simulink model "MX_Gateway"  (Simulink 9.0)
settings: Solver=FixedStepDiscrete  StartTime=0.0  StopTime=100  FixedStep=0.01  RelTol=1e-3
values from neighbouring files (symbols this model uses):
  ACTIVE = Bobcat.Parameter; CreateDefine( ACTIVE                         ,1                          ,'Define' ,'defines.h' )    [defines.m]
  ACTIVE_MODE_ONE = Bobcat.Parameter; CreateDefine( ACTIVE_MODE_ONE                ,19                         ,'Define' ,'defines.h' )    [defines.m]
  AUTHENTICATED = Bobcat.Parameter; CreateDefine( AUTHENTICATED                  ,1                          ,'Define' ,'defines.h' )    [defines.m]
  AUX_EMEA_CONTROLS = Bobcat.Parameter; CreateDefine( AUX_EMEA_CONTROLS  ,1   ,'Define' ,'defines.h' )    [enums.m]
  AUX_FLOW_HIGH = Bobcat.Parameter; CreateDefine( AUX_FLOW_HIGH           ,4  ,'Define' ,'defines.h' )    [enums.m]
  AUX_FLOW_INACTIVE = Bobcat.Parameter; CreateDefine( AUX_FLOW_INACTIVE       ,0  ,'Define' ,'defines.h' )    [enums.m]
  AUX_FLOW_LOW = Bobcat.Parameter; CreateDefine( AUX_FLOW_LOW            ,1  ,'Define' ,'defines.h' )    [enums.m]
  AUX_FLOW_MEDIUM = Bobcat.Parameter; CreateDefine( AUX_FLOW_MEDIUM         ,2  ,'Define' ,'defines.h' )    [enums.m]
  AUX_FLOW_STANDARD = Bobcat.Parameter; CreateDefine( AUX_FLOW_STANDARD       ,3  ,'Define' ,'defines.h' )    [enums.m]
  AUX_NON_FUNCTIONAL = Bobcat.Parameter; CreateDefine( AUX_NON_FUNCTIONAL ,255 ,'Define' ,'defines.h' )    [enums.m]
  BASE_ACTIVE = Bobcat.Parameter; CreateDefine( BASE_ACTIVE                    ,253                        ,'Define' ,'defines.h' )    [defines.m]
  BASE_DETENT = Bobcat.Parameter; CreateDefine( BASE_DETENT                    ,1                          ,'Define' ,'defines.h' )    [defines.m]
  BEEP_NONE = Bobcat.Parameter; CreateDefine( BEEP_NONE                      ,255                        ,'Define' ,'defines.h' )    [defines.m]
  CD_AIR_FILTER_DEBOUNCE_NORMAL = Bobcat.Parameter; CreateAliasCD( CD_AIR_FILTER_DEBOUNCE_NORMAL                        ,uint16(500)                ,'ConstantDataPointer' ,'CONSTANT_DATA' ,'AIR_FILTER_DEBOUNCE_NORMAL')    [cdata.m]    (also in hc12_cdata.m; m4_cdata.m)
  CD_AIR_FILTER_DEBOUNCE_NOT_CONNECTED = Bobcat.Parameter; CreateAliasCD( CD_AIR_FILTER_DEBOUNCE_NOT_CONNECTED                 ,uint16(3000)               ,'ConstantDataPointer' ,'CONSTANT_DATA' ,'AIR_FILTER_DEBOUNCE_NOT_CONNECTED')    [cdata.m]    (also in hc12_cdata.m; m4_cdata.m)
  CD_AIR_FILTER_DEBOUNCE_PLUGGED = Bobcat.Parameter; CreateAliasCD( CD_AIR_FILTER_DEBOUNCE_PLUGGED                       ,uint16(3000)               ,'ConstantDataPointer' ,'CONSTANT_DATA' ,'AIR_FILTER_DEBOUNCE_PLUGGED')    [cdata.m]    (also in hc12_cdata.m; m4_cdata.m)
  CD_AIR_FILTER_FUNCTION = Bobcat.Parameter; CreateAliasCD( CD_AIR_FILTER_FUNCTION                               ,uint8(255)                 ,'ConstantDataPointer' ,'CONSTANT_DATA' ,'AIR_FILTER_FUNCTION')    [cdata.m]    (also in hc12_cdata.m; m4_cdata.m)
  CD_AIR_FILTER_PLUGGED_WEIGHT = Bobcat.Parameter; CreateAliasCD( CD_AIR_FILTER_PLUGGED_WEIGHT                         ,uint8(2)                   ,'ConstantDataPointer' ,'CONSTANT_DATA' ,'AIR_FILTER_PLUGGED_WEIGHT')    [cdata.m]    (also in hc12_cdata.m; m4_cdata.m)
  CD_AIR_FILTER_UNPLUGGED_WEIGHT = Bobcat.Parameter; CreateAliasCD( CD_AIR_FILTER_UNPLUGGED_WEIGHT                       ,uint8(1)                   ,'ConstantDataPointer' ,'CONSTANT_DATA' ,'AIR_FILTER_UNPLUGGED_WEIGHT')    [cdata.m]    (also in hc12_cdata.m; m4_cdata.m)
  CD_AUTOIDLE_ARMBAR_DELAY = Bobcat.Parameter; CreateAliasCD( CD_AUTOIDLE_ARMBAR_DELAY                             ,uint8(2)                   ,'ConstantDataPointer' ,'CONSTANT_DATA' ,'AUTOIDLE_ARMBAR_DELAY')    [cdata.m]    (also in hc12_cdata.m; m4_cdata.m)
  CD_AUTOIDLE_FUNCTION = Bobcat.Parameter; CreateAliasCD( CD_AUTOIDLE_FUNCTION                                 ,uint8(255)                 ,'ConstantDataPointer' ,'CONSTANT_DATA' ,'AUTOIDLE_FUNCTION')    [cdata.m]    (also in hc12_cdata.m; m4_cdata.m)
  CD_AUTOIDLE_IDLE_DELAY = Bobcat.Parameter; CreateAliasCD( CD_AUTOIDLE_IDLE_DELAY                               ,uint8(40)                  ,'ConstantDataPointer' ,'CONSTANT_DATA' ,'AUTOIDLE_IDLE_DELAY')    [cdata.m]    (also in hc12_cdata.m; m4_cdata.m)
  CD_AUTOIDLE_LOW_IDLE_START = Bobcat.Parameter; CreateAliasCD( CD_AUTOIDLE_LOW_IDLE_START                           ,uint8(255)                 ,'ConstantDataPointer' ,'CONSTANT_DATA' ,'AUTOIDLE_LOW_IDLE_START')    [cdata.m]    (also in hc12_cdata.m; m4_cdata.m)
  CD_AUTOIDLE_PRESSURE_OFF = Bobcat.Parameter; CreateAliasCD( CD_AUTOIDLE_PRESSURE_OFF                             ,uint16(300)                ,'ConstantDataPointer' ,'CONSTANT_DATA' ,'AUTOIDLE_PRESSURE_OFF')    [cdata.m]    (also in hc12_cdata.m; m4_cdata.m)
  CD_AUTOIDLE_PRESSURE_ON = Bobcat.Parameter; CreateAliasCD( CD_AUTOIDLE_PRESSURE_ON                              ,uint16(270)                ,'ConstantDataPointer' ,'CONSTANT_DATA' ,'AUTOIDLE_PRESSURE_ON')    [cdata.m]    (also in hc12_cdata.m; m4_cdata.m)
  CD_AUTOSHIFT_FUNCTION = Bobcat.Parameter; CreateAliasCD( CD_AUTOSHIFT_FUNCTION                                ,uint8(255)                 ,'ConstantDataPointer' ,'CONSTANT_DATA' ,'AUTOSHIFT_FUNCTION')    [cdata.m]    (also in hc12_cdata.m; m4_cdata.m)
  CD_AUTOSHIFT_PHIGH1 = Bobcat.Parameter; CreateAliasCD( CD_AUTOSHIFT_PHIGH1                                  ,uint16(205)                ,'ConstantDataPointer' ,'CONSTANT_DATA' ,'AUTOSHIFT_PHIGH1')    [cdata.m]    (also in hc12_cdata.m; m4_cdata.m)
  CD_AUTOSHIFT_PHIGH2 = Bobcat.Parameter; CreateAliasCD( CD_AUTOSHIFT_PHIGH2                                  ,uint16(193)                ,'ConstantDataPointer' ,'CONSTANT_DATA' ,'AUTOSHIFT_PHIGH2')    [cdata.m]    (also in hc12_cdata.m; m4_cdata.m)
  CD_AUTOSHIFT_PHIGH_TEMP1 = Bobcat.Parameter; CreateAliasCD( CD_AUTOSHIFT_PHIGH_TEMP1                             ,int16(70)                  ,'ConstantDataPointer' ,'CONSTANT_DATA' ,'AUTOSHIFT_PHIGH_TEMP1')    [cdata.m]    (also in hc12_cdata.m; m4_cdata.m)
  CD_AUTOSHIFT_PHIGH_TEMP2 = Bobcat.Parameter; CreateAliasCD( CD_AUTOSHIFT_PHIGH_TEMP2                             ,int16(160)                 ,'ConstantDataPointer' ,'CONSTANT_DATA' ,'AUTOSHIFT_PHIGH_TEMP2')    [cdata.m]    (also in hc12_cdata.m; m4_cdata.m)
  CD_AUTOSHIFT_PLOW1 = Bobcat.Parameter; CreateAliasCD( CD_AUTOSHIFT_PLOW1                                   ,uint16(130)                ,'ConstantDataPointer' ,'CONSTANT_DATA' ,'AUTOSHIFT_PLOW1')    [cdata.m]    (also in hc12_cdata.m; m4_cdata.m)
  CD_AUTOSHIFT_PLOW2 = Bobcat.Parameter; CreateAliasCD( CD_AUTOSHIFT_PLOW2                                   ,uint16(100)                ,'ConstantDataPointer' ,'CONSTANT_DATA' ,'AUTOSHIFT_PLOW2')    [cdata.m]    (also in hc12_cdata.m; m4_cdata.m)
  CD_AUTOSHIFT_PLOW_TEMP1 = Bobcat.Parameter; CreateAliasCD( CD_AUTOSHIFT_PLOW_TEMP1                              ,int16(20)                  ,'ConstantDataPointer' ,'CONSTANT_DATA' ,'AUTOSHIFT_PLOW_TEMP1')    [cdata.m]    (also in hc12_cdata.m; m4_cdata.m)
  CD_AUTOSHIFT_PLOW_TEMP2 = Bobcat.Parameter; CreateAliasCD( CD_AUTOSHIFT_PLOW_TEMP2                              ,int16(80)                  ,'ConstantDataPointer' ,'CONSTANT_DATA' ,'AUTOSHIFT_PLOW_TEMP2')    [cdata.m]    (also in hc12_cdata.m; m4_cdata.m)
  CD_AUTOSHIFT_TRAVEL_DELAY = Bobcat.Parameter; CreateAliasCD( CD_AUTOSHIFT_TRAVEL_DELAY                            ,uint8(32)                  ,'ConstantDataPointer' ,'CONSTANT_DATA' ,'AUTOSHIFT_TRAVEL_DELAY')    [cdata.m]    (also in hc12_cdata.m; m4_cdata.m)
  CD_AUX2_CRACKPOINT_CURRENT = Bobcat.Parameter; CreateAliasCD( CD_AUX2_CRACKPOINT_CURRENT                           ,fi(0,0,16,12,'hex','0c28') ,'ConstantDataPointer' ,'CONSTANT_DATA' ,'AUX2_CRACKPOINT_CURRENT')    [cdata.m]    (also in hc12_cdata.m; m4_cdata.m)
  CD_AUX2_FLOW_LOW_FUNCTION = Bobcat.Parameter; CreateAliasCD( CD_AUX2_FLOW_LOW_FUNCTION                            ,uint8(255)                 ,'ConstantDataPointer' ,'CONSTANT_DATA' ,'AUX2_FLOW_LOW_FUNCTION')    [cdata.m]    (also in hc12_cdata.m; m4_cdata.m)
  CD_AUX2_FLOW_MED_FUNCTION = Bobcat.Parameter; CreateAliasCD( CD_AUX2_FLOW_MED_FUNCTION                            ,uint8(255)                 ,'ConstantDataPointer' ,'CONSTANT_DATA' ,'AUX2_FLOW_MED_FUNCTION')    [cdata.m]    (also in hc12_cdata.m; m4_cdata.m)
  CD_AUX2_HI_FLOW_PERCENT = Bobcat.Parameter; CreateAliasCD( CD_AUX2_HI_FLOW_PERCENT                              ,fi(0,0,16,16,'hex','ffff') ,'ConstantDataPointer' ,'CONSTANT_DATA' ,'AUX2_HI_FLOW_PERCENT')    [cdata.m]    (also in hc12_cdata.m; m4_cdata.m)
  CD_AUX2_LOW_FLOW_PERCENT = Bobcat.Parameter; CreateAliasCD( CD_AUX2_LOW_FLOW_PERCENT                             ,fi(0,0,16,16,'hex','7333') ,'ConstantDataPointer' ,'CONSTANT_DATA' ,'AUX2_LOW_FLOW_PERCENT')    [cdata.m]    (also in hc12_cdata.m; m4_cdata.m)
  CD_AUX2_MAX_FLOW_CURRENT = Bobcat.Parameter; CreateAliasCD( CD_AUX2_MAX_FLOW_CURRENT                             ,fi(0,0,16,12,'hex','14cc') ,'ConstantDataPointer' ,'CONSTANT_DATA' ,'AUX2_MAX_FLOW_CURRENT')    [cdata.m]    (also in hc12_cdata.m; m4_cdata.m)
  CD_AUX2_MED_FLOW_PERCENT = Bobcat.Parameter; CreateAliasCD( CD_AUX2_MED_FLOW_PERCENT                             ,fi(0,0,16,16,'hex','9999') ,'ConstantDataPointer' ,'CONSTANT_DATA' ,'AUX2_MED_FLOW_PERCENT')    [cdata.m]    (also in hc12_cdata.m; m4_cdata.m)
  CD_AUX_CRACKPOINT_CURRENT = Bobcat.Parameter; CreateAliasCD( CD_AUX_CRACKPOINT_CURRENT                            ,fi(0,0,16,12,'hex','0000') ,'ConstantDataPointer' ,'CONSTANT_DATA' ,'AUX_CRACKPOINT_CURRENT')    [cdata.m]    (also in hc12_cdata.m; m4_cdata.m)
  CD_AUX_DETENT_FLASH_TIME = Bobcat.Parameter; CreateAliasCD( CD_AUX_DETENT_FLASH_TIME                             ,uint16(72)                 ,'ConstantDataPointer' ,'CONSTANT_DATA' ,'AUX_DETENT_FLASH_TIME')    [cdata.m]    (also in hc12_cdata.m; m4_cdata.m)
  CD_AUX_DETENT_HOLD_TIME = Bobcat.Parameter; CreateAliasCD( CD_AUX_DETENT_HOLD_TIME                              ,uint16(100)                ,'ConstantDataPointer' ,'CONSTANT_DATA' ,'AUX_DETENT_HOLD_TIME')    [cdata.m]    (also in hc12_cdata.m; m4_cdata.m)
  CD_AUX_FULL_ON_AUX1 = Bobcat.Parameter; CreateAliasCD( CD_AUX_FULL_ON_AUX1                                  ,fi(0,0,16,12,'hex','10cd') ,'ConstantDataPointer' ,'CONSTANT_DATA' ,'AUX_FULL_ON_AUX1')    [cdata.m]    (also in hc12_cdata.m; m4_cdata.m)
  CD_AUX_FULL_ON_AUX2 = Bobcat.Parameter; CreateAliasCD( CD_AUX_FULL_ON_AUX2                                  ,fi(0,0,16,12,'hex','139d') ,'ConstantDataPointer' ,'CONSTANT_DATA' ,'AUX_FULL_ON_AUX2')    [cdata.m]    (also in hc12_cdata.m; m4_cdata.m)
  CD_AUX_FULL_ON_AUX3 = Bobcat.Parameter; CreateAliasCD( CD_AUX_FULL_ON_AUX3                                  ,fi(0,0,16,12,'hex','17d7') ,'ConstantDataPointer' ,'CONSTANT_DATA' ,'AUX_FULL_ON_AUX3')    [cdata.m]    (also in hc12_cdata.m; m4_cdata.m)
  CD_AUX_FUNCTION = Bobcat.Parameter; CreateAliasCD( CD_AUX_FUNCTION                                      ,uint8(0)                   ,'ConstantDataPointer' ,'CONSTANT_DATA' ,'AUX_FUNCTION')    [cdata.m]    (also in hc12_cdata.m; m4_cdata.m)
  CD_AUX_RATE_LIMIT_CRACKPOINT = Bobcat.Parameter; CreateAliasCD( CD_AUX_RATE_LIMIT_CRACKPOINT                         ,fi(0,0,16,12,'hex','0800') ,'ConstantDataPointer' ,'CONSTANT_DATA' ,'AUX_RATE_LIMIT_CRACKPOINT')    [cdata.m]    (also in hc12_cdata.m; m4_cdata.m)
  CD_AUX_RATE_LIMIT_CRACKPOINT_RATE = Bobcat.Parameter; CreateAliasCD( CD_AUX_RATE_LIMIT_CRACKPOINT_RATE                    ,fi(0,1,32,12,'int',102400) ,'ConstantDataPointer' ,'CONSTANT_DATA' ,'AUX_RATE_LIMIT_CRACKPOINT_RATE')    [cdata.m]    (also in hc12_cdata.m; m4_cdata.m)
  CD_AUX_RATE_LIMIT_FUNCTION = Bobcat.Parameter; CreateAliasCD( CD_AUX_RATE_LIMIT_FUNCTION                           ,uint8(255)                 ,'ConstantDataPointer' ,'CONSTANT_DATA' ,'AUX_RATE_LIMIT_FUNCTION')    [cdata.m]    (also in hc12_cdata.m; m4_cdata.m)
  CD_AUX_RATE_LIMIT_MAX_ENG_SPD = Bobcat.Parameter; CreateAliasCD( CD_AUX_RATE_LIMIT_MAX_ENG_SPD                        ,uint16(1600)               ,'ConstantDataPointer' ,'CONSTANT_DATA' ,'AUX_RATE_LIMIT_MAX_ENG_SPD')    [cdata.m]    (also in hc12_cdata.m; m4_cdata.m)
  CD_AUX_RATE_LIMIT_MIN_ENG_SPD = Bobcat.Parameter; CreateAliasCD( CD_AUX_RATE_LIMIT_MIN_ENG_SPD                        ,uint16(100)                ,'ConstantDataPointer' ,'CONSTANT_DATA' ,'AUX_RATE_LIMIT_MIN_ENG_SPD')    [cdata.m]    (also in hc12_cdata.m; m4_cdata.m)
  CD_AUX_RATE_LIMIT_NORMAL_RATE = Bobcat.Parameter; CreateAliasCD( CD_AUX_RATE_LIMIT_NORMAL_RATE                        ,fi(0,1,32,12,'int',2048)   ,'ConstantDataPointer' ,'CONSTANT_DATA' ,'AUX_RATE_LIMIT_NORMAL_RATE')    [cdata.m]    (also in hc12_cdata.m; m4_cdata.m)
  CD_AUXPOT_CAL_DEADBAND_HIGH = Bobcat.Parameter; CreateAliasCD( CD_AUXPOT_CAL_DEADBAND_HIGH                          ,uint16(574)                ,'ConstantDataPointer' ,'CONSTANT_DATA' ,'AUXPOT_CAL_DEADBAND_HIGH')    [cdata.m]    (also in hc12_cdata.m; m4_cdata.m)
  CD_AUXPOT_CAL_DEADBAND_LOW = Bobcat.Parameter; CreateAliasCD( CD_AUXPOT_CAL_DEADBAND_LOW                           ,uint16(424)                ,'ConstantDataPointer' ,'CONSTANT_DATA' ,'AUXPOT_CAL_DEADBAND_LOW')    [cdata.m]    (also in hc12_cdata.m; m4_cdata.m)
  CD_AUXPOT_CENTER_RATIO = Bobcat.Parameter; CreateAliasCD( CD_AUXPOT_CENTER_RATIO                               ,uint16(500)                ,'ConstantDataPointer' ,'CONSTANT_DATA' ,'AUXPOT_CENTER_RATIO')    [cdata.m]    (also in hc12_cdata.m; m4_cdata.m)
  CD_AUXPOT_DEADBAND = Bobcat.Parameter; CreateAliasCD( CD_AUXPOT_DEADBAND                                   ,uint8(38)                  ,'ConstantDataPointer' ,'CONSTANT_DATA' ,'AUXPOT_DEADBAND')    [cdata.m]    (also in hc12_cdata.m; m4_cdata.m)
  CD_AUXPOT_FUNCTION = Bobcat.Parameter; CreateAliasCD( CD_AUXPOT_FUNCTION                                   ,uint8(0)                   ,'ConstantDataPointer' ,'CONSTANT_DATA' ,'AUXPOT_FUNCTION')    [cdata.m]    (also in hc12_cdata.m; m4_cdata.m)
  CD_AUXPOT_RATIO_GAIN = Bobcat.Parameter; CreateAliasCD( CD_AUXPOT_RATIO_GAIN                                 ,uint16(1000)               ,'ConstantDataPointer' ,'CONSTANT_DATA' ,'AUXPOT_RATIO_GAIN')    [cdata.m]    (also in hc12_cdata.m; m4_cdata.m)
  CD_CAN_THROTTLE_FUNCTION = Bobcat.Parameter; CreateAliasCD( CD_CAN_THROTTLE_FUNCTION                             ,uint8(255)                 ,'ConstantDataPointer' ,'CONSTANT_DATA' ,'CAN_THROTTLE_FUNCTION')    [cdata.m]    (also in hc12_cdata.m; m4_cdata.m)
  CD_CAN_THROTTLE_REVERSE = Bobcat.Parameter; CreateAliasCD( CD_CAN_THROTTLE_REVERSE                              ,uint8(255)                 ,'ConstantDataPointer' ,'CONSTANT_DATA' ,'CAN_THROTTLE_REVERSE')    [cdata.m]    (also in hc12_cdata.m; m4_cdata.m)
  CD_CONSOLE_DEBOUNCE_ABSENT = Bobcat.Parameter; CreateAliasCD( CD_CONSOLE_DEBOUNCE_ABSENT                           ,uint16(12)                 ,'ConstantDataPointer' ,'CONSTANT_DATA' ,'CONSOLE_DEBOUNCE_ABSENT')    [cdata.m]    (also in hc12_cdata.m; m4_cdata.m)
  CD_CONSOLE_DEBOUNCE_OORH = Bobcat.Parameter; CreateAliasCD( CD_CONSOLE_DEBOUNCE_OORH                             ,uint16(12)                 ,'ConstantDataPointer' ,'CONSTANT_DATA' ,'CONSOLE_DEBOUNCE_OORH')    [cdata.m]    (also in hc12_cdata.m; m4_cdata.m)
  CD_CONSOLE_DEBOUNCE_OORL = Bobcat.Parameter; CreateAliasCD( CD_CONSOLE_DEBOUNCE_OORL                             ,uint16(12)                 ,'ConstantDataPointer' ,'CONSTANT_DATA' ,'CONSOLE_DEBOUNCE_OORL')    [cdata.m]    (also in hc12_cdata.m; m4_cdata.m)
  CD_CONSOLE_DEBOUNCE_PRESENT = Bobcat.Parameter; CreateAliasCD( CD_CONSOLE_DEBOUNCE_PRESENT                          ,uint16(12)                 ,'ConstantDataPointer' ,'CONSTANT_DATA' ,'CONSOLE_DEBOUNCE_PRESENT')    [cdata.m]    (also in hc12_cdata.m; m4_cdata.m)
  CD_CONSOLE_FUNCTION = Bobcat.Parameter; CreateAliasCD( CD_CONSOLE_FUNCTION                                  ,uint8(0)                   ,'ConstantDataPointer' ,'CONSTANT_DATA' ,'CONSOLE_FUNCTION')    [cdata.m]    (also in hc12_cdata.m; m4_cdata.m)
  CD_CONSOLE_OORH = Bobcat.Parameter; CreateAliasCD( CD_CONSOLE_OORH                                      ,uint16(740)                ,'ConstantDataPointer' ,'CONSTANT_DATA' ,'CONSOLE_OORH')    [cdata.m]    (also in hc12_cdata.m; m4_cdata.m)
  CD_CONSOLE_OORL = Bobcat.Parameter; CreateAliasCD( CD_CONSOLE_OORL                                      ,uint16(10)                 ,'ConstantDataPointer' ,'CONSTANT_DATA' ,'CONSOLE_OORL')    [cdata.m]    (also in hc12_cdata.m; m4_cdata.m)
  CD_CONSOLE_PRESENT_HIGH = Bobcat.Parameter; CreateAliasCD( CD_CONSOLE_PRESENT_HIGH                              ,uint16(515)                ,'ConstantDataPointer' ,'CONSTANT_DATA' ,'CONSOLE_PRESENT_HIGH')    [cdata.m]    (also in hc12_cdata.m; m4_cdata.m)
  CD_CONSOLE_PRESENT_LOW = Bobcat.Parameter; CreateAliasCD( CD_CONSOLE_PRESENT_LOW                               ,uint16(375)                ,'ConstantDataPointer' ,'CONSTANT_DATA' ,'CONSOLE_PRESENT_LOW')    [cdata.m]    (also in hc12_cdata.m; m4_cdata.m)
  CD_DELUXE_GEN5_5_FUNCTION = Bobcat.Parameter; CreateAliasCD( CD_DELUXE_GEN5_5_FUNCTION                            ,uint8(255)                 ,'ConstantDataPointer' ,'CONSTANT_DATA' ,'DELUXE_GEN5_5_FUNCTION')    [cdata.m]    (also in hc12_cdata.m; m4_cdata.m)
  CD_DELUXE_GEN5_FUNCTION = Bobcat.Parameter; CreateAliasCD( CD_DELUXE_GEN5_FUNCTION                              ,uint8(255)                 ,'ConstantDataPointer' ,'CONSTANT_DATA' ,'DELUXE_GEN5_FUNCTION')    [cdata.m]    (also in hc12_cdata.m; m4_cdata.m)
  CD_DERATE_ENGINE_SPEED_DEBOUNCE_HIGH = Bobcat.Parameter; CreateAliasCD( CD_DERATE_ENGINE_SPEED_DEBOUNCE_HIGH                 ,uint16(200)                ,'ConstantDataPointer' ,'CONSTANT_DATA' ,'DERATE_ENGINE_SPEED_DEBOUNCE_HIGH')    [cdata.m]    (also in hc12_cdata.m; m4_cdata.m)
  CD_DERATE_ENGINE_SPEED_DEBOUNCE_SHUTDOWN = Bobcat.Parameter; CreateAliasCD( CD_DERATE_ENGINE_SPEED_DEBOUNCE_SHUTDOWN             ,uint16(1000)               ,'ConstantDataPointer' ,'CONSTANT_DATA' ,'DERATE_ENGINE_SPEED_DEBOUNCE_SHUTDOWN')    [cdata.m]    (also in hc12_cdata.m; m4_cdata.m)
  CD_DERATE_ENGINE_SPEED_HIGH_OFFSET = Bobcat.Parameter; CreateAliasCD( CD_DERATE_ENGINE_SPEED_HIGH_OFFSET                   ,uint16(200)                ,'ConstantDataPointer' ,'CONSTANT_DATA' ,'DERATE_ENGINE_SPEED_HIGH_OFFSET')    [cdata.m]    (also in hc12_cdata.m; m4_cdata.m)
  CD_DERATE_METHOD = Bobcat.Parameter; CreateAliasCD( CD_DERATE_METHOD                                     ,uint8(0)                   ,'ConstantDataPointer' ,'CONSTANT_DATA' ,'DERATE_METHOD')    [cdata.m]    (also in hc12_cdata.m; m4_cdata.m)
  CD_DUMP_VALVE_FUNCTION = Bobcat.Parameter; CreateAliasCD( CD_DUMP_VALVE_FUNCTION                               ,uint8(255)                 ,'ConstantDataPointer' ,'CONSTANT_DATA' ,'DUMP_VALVE_FUNCTION')    [cdata.m]    (also in hc12_cdata.m; m4_cdata.m)
  CD_DUMP_VALVE_ON_CURRENT = Bobcat.Parameter; CreateAliasCD( CD_DUMP_VALVE_ON_CURRENT                             ,fi(0,0,16,12,'hex','ffff') ,'ConstantDataPointer' ,'CONSTANT_DATA' ,'DUMP_VALVE_ON_CURRENT')    [cdata.m]    (also in hc12_cdata.m; m4_cdata.m)
  CD_ECO_MODE_THROTTLE_REDUCTION = Bobcat.Parameter; CreateAliasCD( CD_ECO_MODE_THROTTLE_REDUCTION                       ,uint16(850)                ,'ConstantDataPointer' ,'CONSTANT_DATA' ,'ECO_MODE_THROTTLE_REDUCTION')    [cdata.m]    (also in hc12_cdata.m; m4_cdata.m)
  CD_ECT_FILTER_GAIN = Bobcat.Parameter; CreateAliasCD( CD_ECT_FILTER_GAIN                                   ,uint8(6)                   ,'ConstantDataPointer' ,'CONSTANT_DATA' ,'ECT_FILTER_GAIN')    [cdata.m]    (also in hc12_cdata.m; m4_cdata.m)
  CD_ECU_FUNCTION = Bobcat.Parameter; CreateAliasCD( CD_ECU_FUNCTION                                      ,uint8(255)                 ,'ConstantDataPointer' ,'CONSTANT_DATA' ,'ECU_FUNCTION')    [cdata.m]    (also in hc12_cdata.m; m4_cdata.m)
  CD_ECU_HOLD_DEBOUNCE_ERROR_ON = Bobcat.Parameter; CreateAliasCD( CD_ECU_HOLD_DEBOUNCE_ERROR_ON                        ,uint16(20)                 ,'ConstantDataPointer' ,'CONSTANT_DATA' ,'ECU_HOLD_DEBOUNCE_ERROR_ON')    [cdata.m]    (also in hc12_cdata.m; m4_cdata.m)
  CD_EMEA_CONTROLS_HOLD_TIME = Bobcat.Parameter; CreateAliasCD( CD_EMEA_CONTROLS_HOLD_TIME                           ,uint16(30)                 ,'ConstantDataPointer' ,'CONSTANT_DATA' ,'EMEA_CONTROLS_HOLD_TIME')    [cdata.m]    (also in hc12_cdata.m; m4_cdata.m)
  CD_EMEA_CONTROLS_OPTION_A = Bobcat.Parameter; CreateAliasCD( CD_EMEA_CONTROLS_OPTION_A                            ,uint8(255)                 ,'ConstantDataPointer' ,'CONSTANT_DATA' ,'EMEA_CONTROLS_OPTION_A')    [cdata.m]    (also in hc12_cdata.m; m4_cdata.m)
  CD_EMEA_CONTROLS_OPTION_C = Bobcat.Parameter; CreateAliasCD( CD_EMEA_CONTROLS_OPTION_C                            ,uint8(255)                 ,'ConstantDataPointer' ,'CONSTANT_DATA' ,'EMEA_CONTROLS_OPTION_C')    [cdata.m]    (also in hc12_cdata.m; m4_cdata.m)
  CD_EMEA_CONTROLS_REVERSE = Bobcat.Parameter; CreateAliasCD( CD_EMEA_CONTROLS_REVERSE                             ,uint8(255)                 ,'ConstantDataPointer' ,'CONSTANT_DATA' ,'EMEA_CONTROLS_REVERSE')    [cdata.m]    (also in hc12_cdata.m; m4_cdata.m)
  CD_EMEA_ELECTRIC_VERSION = Bobcat.Parameter; CreateAliasCD( CD_EMEA_ELECTRIC_VERSION                             ,uint8(0)                   ,'ConstantDataPointer' ,'CONSTANT_DATA' ,'EMEA_ELECTRIC_VERSION')    [cdata.m]    (also in hc12_cdata.m; m4_cdata.m)
  CD_EMEA_POT_CAL_DEADBAND_HIGH = Bobcat.Parameter; CreateAliasCD( CD_EMEA_POT_CAL_DEADBAND_HIGH                        ,uint16(574)                ,'ConstantDataPointer' ,'CONSTANT_DATA' ,'EMEA_POT_CAL_DEADBAND_HIGH')    [cdata.m]    (also in hc12_cdata.m; m4_cdata.m)
  CD_EMEA_POT_CAL_DEADBAND_LOW = Bobcat.Parameter; CreateAliasCD( CD_EMEA_POT_CAL_DEADBAND_LOW                         ,uint16(424)                ,'ConstantDataPointer' ,'CONSTANT_DATA' ,'EMEA_POT_CAL_DEADBAND_LOW')    [cdata.m]    (also in hc12_cdata.m; m4_cdata.m)
  CD_ENGINE_RPM_FACTOR = Bobcat.Parameter; CreateAliasCD( CD_ENGINE_RPM_FACTOR                                 ,uint8(35)                  ,'ConstantDataPointer' ,'CONSTANT_DATA' ,'ENGINE_RPM_FACTOR')    [cdata.m]    (also in hc12_cdata.m; m4_cdata.m)
  CD_ENGINE_SPEED_FILTER_FAST_VALUE = Bobcat.Parameter; CreateAliasCD( CD_ENGINE_SPEED_FILTER_FAST_VALUE                    ,uint8(1)                   ,'ConstantDataPointer' ,'CONSTANT_DATA' ,'ENGINE_SPEED_FILTER_FAST_VALUE')    [cdata.m]    (also in hc12_cdata.m; m4_cdata.m)
  CD_ENGINE_SPEED_FILTER_SLOW_VALUE = Bobcat.Parameter; CreateAliasCD( CD_ENGINE_SPEED_FILTER_SLOW_VALUE                    ,uint8(4)                   ,'ConstantDataPointer' ,'CONSTANT_DATA' ,'ENGINE_SPEED_FILTER_SLOW_VALUE')    [cdata.m]    (also in hc12_cdata.m; m4_cdata.m)
  CD_ENGINE_SPEED_RUNNING = Bobcat.Parameter; CreateAliasCD( CD_ENGINE_SPEED_RUNNING                              ,uint16(800)                ,'ConstantDataPointer' ,'CONSTANT_DATA' ,'ENGINE_SPEED_RUNNING')    [cdata.m]    (also in hc12_cdata.m; m4_cdata.m)
  CD_ENGINE_START_DELAY_DEBOUNCE_RPM_NO_SIGNAL = Bobcat.Parameter; CreateAliasCD( CD_ENGINE_START_DELAY_DEBOUNCE_RPM_NO_SIGNAL         ,uint16(0)                  ,'ConstantDataPointer' ,'CONSTANT_DATA' ,'ENGINE_START_DELAY_DEBOUNCE_RPM_NO_SIGNAL')    [cdata.m]    (also in hc12_cdata.m; m4_cdata.m)
  CD_ENGINE_START_DELAY_OFF_TIME = Bobcat.Parameter; CreateAliasCD( CD_ENGINE_START_DELAY_OFF_TIME                       ,uint16(0)                  ,'ConstantDataPointer' ,'CONSTANT_DATA' ,'ENGINE_START_DELAY_OFF_TIME')    [cdata.m]    (also in hc12_cdata.m; m4_cdata.m)
  CD_ENGINE_START_DELAY_RPM_NO_SIGNAL = Bobcat.Parameter; CreateAliasCD( CD_ENGINE_START_DELAY_RPM_NO_SIGNAL                  ,uint16(0)                  ,'ConstantDataPointer' ,'CONSTANT_DATA' ,'ENGINE_START_DELAY_RPM_NO_SIGNAL')    [cdata.m]    (also in hc12_cdata.m; m4_cdata.m)
  CD_EOP_DEBOUNCE_EXTREMELY_LOW = Bobcat.Parameter; CreateAliasCD( CD_EOP_DEBOUNCE_EXTREMELY_LOW                        ,uint16(50)                 ,'ConstantDataPointer' ,'CONSTANT_DATA' ,'EOP_DEBOUNCE_EXTREMELY_LOW')    [cdata.m]    (also in hc12_cdata.m; m4_cdata.m)
  CD_EOP_DEBOUNCE_NORMAL = Bobcat.Parameter; CreateAliasCD( CD_EOP_DEBOUNCE_NORMAL                               ,uint16(50)                 ,'ConstantDataPointer' ,'CONSTANT_DATA' ,'EOP_DEBOUNCE_NORMAL')    [cdata.m]    (also in hc12_cdata.m; m4_cdata.m)
  CD_EOP_DEBOUNCE_SHUTDOWN = Bobcat.Parameter; CreateAliasCD( CD_EOP_DEBOUNCE_SHUTDOWN                             ,uint16(100)                ,'ConstantDataPointer' ,'CONSTANT_DATA' ,'EOP_DEBOUNCE_SHUTDOWN')    [cdata.m]    (also in hc12_cdata.m; m4_cdata.m)
  CD_EOP_FILTER_VALUE = Bobcat.Parameter; CreateAliasCD( CD_EOP_FILTER_VALUE                                  ,uint8(3)                   ,'ConstantDataPointer' ,'CONSTANT_DATA' ,'EOP_FILTER_VALUE')    [cdata.m]    (also in hc12_cdata.m; m4_cdata.m)
  CD_EOP_FUNCTION = Bobcat.Parameter; CreateAliasCD( CD_EOP_FUNCTION                                      ,uint8(1)                   ,'ConstantDataPointer' ,'CONSTANT_DATA' ,'EOP_FUNCTION')    [cdata.m]    (also in hc12_cdata.m; m4_cdata.m)
  CD_EOP_SWITCH_GAUGE_VALUE = Bobcat.Parameter; CreateAliasCD( CD_EOP_SWITCH_GAUGE_VALUE                            ,uint16(125)                ,'ConstantDataPointer' ,'CONSTANT_DATA' ,'EOP_SWITCH_GAUGE_VALUE')    [cdata.m]    (also in hc12_cdata.m; m4_cdata.m)
  CD_EOP_SWITCH_LOW = Bobcat.Parameter; CreateAliasCD( CD_EOP_SWITCH_LOW                                    ,uint16(50)                 ,'ConstantDataPointer' ,'CONSTANT_DATA' ,'EOP_SWITCH_LOW')    [cdata.m]    (also in hc12_cdata.m; m4_cdata.m)
  CD_EOP_SWITCH_PRESSURE_VALUE = Bobcat.Parameter; CreateAliasCD( CD_EOP_SWITCH_PRESSURE_VALUE                         ,uint16(40)                 ,'ConstantDataPointer' ,'CONSTANT_DATA' ,'EOP_SWITCH_PRESSURE_VALUE')    [cdata.m]    (also in hc12_cdata.m; m4_cdata.m)
  CD_EOT_DEBOUNCE_EXTREMELY_LOW = Bobcat.Parameter; CreateAliasCD( CD_EOT_DEBOUNCE_EXTREMELY_LOW                        ,uint16(2)                  ,'ConstantDataPointer' ,'CONSTANT_DATA' ,'EOT_DEBOUNCE_EXTREMELY_LOW')    [cdata.m]    (also in hc12_cdata.m; m4_cdata.m)
  CD_EOT_DEBOUNCE_LOW = Bobcat.Parameter; CreateAliasCD( CD_EOT_DEBOUNCE_LOW                                  ,uint16(2)                  ,'ConstantDataPointer' ,'CONSTANT_DATA' ,'EOT_DEBOUNCE_LOW')    [cdata.m]    (also in hc12_cdata.m; m4_cdata.m)
  CD_EOT_DEBOUNCE_NORMAL = Bobcat.Parameter; CreateAliasCD( CD_EOT_DEBOUNCE_NORMAL                               ,uint16(2)                  ,'ConstantDataPointer' ,'CONSTANT_DATA' ,'EOT_DEBOUNCE_NORMAL')    [cdata.m]    (also in hc12_cdata.m; m4_cdata.m)
  CD_EOT_EXTREMELY_LOW = Bobcat.Parameter; CreateAliasCD( CD_EOT_EXTREMELY_LOW                                 ,uint16(81)                 ,'ConstantDataPointer' ,'CONSTANT_DATA' ,'EOT_EXTREMELY_LOW')    [cdata.m]    (also in hc12_cdata.m; m4_cdata.m)
  CD_EOT_FUNCTION = Bobcat.Parameter; CreateAliasCD( CD_EOT_FUNCTION                                      ,uint8(255)                 ,'ConstantDataPointer' ,'CONSTANT_DATA' ,'EOT_FUNCTION')    [cdata.m]    (also in hc12_cdata.m; m4_cdata.m)
  CD_EOT_LOW = Bobcat.Parameter; CreateAliasCD( CD_EOT_LOW                                           ,uint16(90)                 ,'ConstantDataPointer' ,'CONSTANT_DATA' ,'EOT_LOW')    [cdata.m]    (also in hc12_cdata.m; m4_cdata.m)
  CD_EOT_TEMPERATURE_HYSTERESIS = Bobcat.Parameter; CreateAliasCD( CD_EOT_TEMPERATURE_HYSTERESIS                        ,uint16(2)                  ,'ConstantDataPointer' ,'CONSTANT_DATA' ,'EOT_TEMPERATURE_HYSTERESIS')    [cdata.m]    (also in hc12_cdata.m; m4_cdata.m)
  CD_FAST_TRAVEL_FUNCTION = Bobcat.Parameter; CreateAliasCD( CD_FAST_TRAVEL_FUNCTION                              ,uint8(255)                 ,'ConstantDataPointer' ,'CONSTANT_DATA' ,'FAST_TRAVEL_FUNCTION')    [cdata.m]    (also in hc12_cdata.m; m4_cdata.m)
  CD_FAST_TRAVEL_THROTTLE_DELAY = Bobcat.Parameter; CreateAliasCD( CD_FAST_TRAVEL_THROTTLE_DELAY                        ,uint16(10)                 ,'ConstantDataPointer' ,'CONSTANT_DATA' ,'FAST_TRAVEL_THROTTLE_DELAY')    [cdata.m]    (also in hc12_cdata.m; m4_cdata.m)
  CD_FAST_TRAVEL_THROTTLE_LIMIT = Bobcat.Parameter; CreateAliasCD( CD_FAST_TRAVEL_THROTTLE_LIMIT                        ,uint16(1000)               ,'ConstantDataPointer' ,'CONSTANT_DATA' ,'FAST_TRAVEL_THROTTLE_LIMIT')    [cdata.m]    (also in hc12_cdata.m; m4_cdata.m)
  CD_FLYWHEEL_TEETH = Bobcat.Parameter; CreateAliasCD( CD_FLYWHEEL_TEETH                                    ,uint8(83)                  ,'ConstantDataPointer' ,'CONSTANT_DATA' ,'FLYWHEEL_TEETH')    [cdata.m]    (also in hc12_cdata.m; m4_cdata.m)
  CD_FUEL_LEVEL_FILTER_FAST_VALUE = Bobcat.Parameter; CreateAliasCD( CD_FUEL_LEVEL_FILTER_FAST_VALUE                      ,uint8(5)                   ,'ConstantDataPointer' ,'CONSTANT_DATA' ,'FUEL_LEVEL_FILTER_FAST_VALUE')    [cdata.m]    (also in hc12_cdata.m; m4_cdata.m)
  CD_FUEL_LEVEL_FILTER_SLOW_VALUE = Bobcat.Parameter; CreateAliasCD( CD_FUEL_LEVEL_FILTER_SLOW_VALUE                      ,uint8(10)                  ,'ConstantDataPointer' ,'CONSTANT_DATA' ,'FUEL_LEVEL_FILTER_SLOW_VALUE')    [cdata.m]    (also in hc12_cdata.m; m4_cdata.m)
  CD_FUEL_PRIMARY_FEEDBACK_FUNCTION = Bobcat.Parameter; CreateAliasCD( CD_FUEL_PRIMARY_FEEDBACK_FUNCTION                    ,uint8(0)                   ,'ConstantDataPointer' ,'CONSTANT_DATA' ,'FUEL_PRIMARY_FEEDBACK_FUNCTION')    [cdata.m]    (also in hc12_cdata.m; m4_cdata.m)
  ... (+246 more)
undefined symbols (not defined in the file or in its folder): sigbuilder_block
undefined symbols (not defined in the file): CD_FUEL_RESOLUTION_TIMER
libraries: simulink x186, MX_Library x107
(part 17 of 48)

== system MX_Gateway/MX_Gtwy_Control/Outputs/Two_Speed ==
  state_Engine : Inport
  state_ECT : Inport  Port=2
  state_HOT : Inport  Port=3
  state_Console : Inport  Port=4
  state_LoadSense : Inport  Port=5
  flt_HOT : Inport  Port=6
  calc_Vbattery : Inport  Port=7
  state_Vbattery : Inport  Port=8
  bar_LoadSense : Inport  Port=9
  state_System : Inport  Port=10
  afbk_TwoSpeed : Inport  Port=11
  din_TwoSpeedSwitch : Inport  Port=12
  adin_TravelPressureSwitch : Inport  Port=13
  state_HydEnable : Inport  Port=14
  Compare To Constant : simulink/Logic and Bit Operations/Compare To Constant  relop=>  const=512  OutDataTypeStr=boolean
  CompareToConstant : simulink/Logic and Bit Operations/Compare To Constant  relop=~=  const=NON_FUNCTIONAL  OutDataTypeStr=boolean  ZeroCross=off
  CompareToConstant1 : simulink/Logic and Bit Operations/Compare To Constant  relop===  const=SYS_NORMAL  OutDataTypeStr=boolean  ZeroCross=off
  Constant : Constant  Value=CD_TWOSPEED_FUNCTION  OutDataTypeStr=uint8
  Data Type Conversion3 : DataTypeConversion  OutDataTypeStr=uint16
  Logical Operator1 : Logic  AllPortsSameDT=off  OutDataTypeStr=boolean
  Two_Speed : Subsystem
  state_TwoSpeed : Outport
  state_TwoSpeedIcon : Outport  Port=2
  duty_TwoSpeed : Outport  Port=3
  wires:
  state_Engine/1 -> Two_Speed/1(state_Engine)
  state_ECT/1 -> Two_Speed/2(state_ECT)
  Constant/1 -> CompareToConstant/1
  CompareToConstant/1 -> Logical Operator1/1
  Two_Speed/1(state_TwoSpeed) -> state_TwoSpeed/1
  Two_Speed/2(state_TwoSpeedIcon) -> state_TwoSpeedIcon/1
  state_LoadSense/1 -> Two_Speed/6(state_LoadSense)
  flt_HOT/1 -> Two_Speed/7(flt_HOT)
  state_System/1 -> CompareToConstant1/1
  CompareToConstant1/1 -> Logical Operator1/2
  state_HOT/1 -> Two_Speed/3(state_HOT)
  state_HydEnable/1 -> Two_Speed/4(state_HydEnable)
  state_Console/1 -> Two_Speed/5(state_Console)
  afbk_TwoSpeed/1 -> Two_Speed/9(afbk_TwoSpeed)
  state_Vbattery/1 -> Two_Speed/10(state_Vbattery)
  calc_Vbattery/1 -> Two_Speed/11(calc_Vbattery)
  Two_Speed/3(duty_TwoSpeed) -> duty_TwoSpeed/1
  adin_TravelPressureSwitch/1 -> Compare To Constant/1
  Logical Operator1/1 -> Two_Speed/Enable
  Compare To Constant/1 -> Two_Speed/8(din_TravelPressureSwitch)
  bar_LoadSense/1 -> Data Type Conversion3/1
  Data Type Conversion3/1 -> Two_Speed/12(bar_LoadSense)
  din_TwoSpeedSwitch/1 -> Two_Speed/13(din_TwoSpeedSwitch)

== system MX_Gateway/MX_Gtwy_Control/PanelInterface/AutoIdle_Ind ==
  state_AutoIdleEnable : Inport
  AutoIdle_Indicator : Stateflow chart  TreatAsAtomicUnit=on
  ind_AutoIdle : Outport
  wires:
  AutoIdle_Indicator/1(ind_AutoIdle) -> ind_AutoIdle/1
  state_AutoIdleEnable/1 -> AutoIdle_Indicator/1(state_AutoIdleEnable)

== system MX_Gateway/MX_Gtwy_Control/PanelInterface/Aux_Ind ==
  state_AuxEnable : Inport
  state_DetentEnable : Inport  Port=2
  state_Detent : Inport  Port=3
  flag_AuxEnableFlash : Inport  Port=4
  Aux1_Indicator : Stateflow chart  TreatAsAtomicUnit=on
  Aux2_Indicator : Stateflow chart  TreatAsAtomicUnit=on
  ind_Aux1 : Outport
  ind_Aux2 : Outport  Port=2
  wires:
  state_DetentEnable/1 -> Aux2_Indicator/1(state_DetentEnable), Aux1_Indicator/4(state_DetentEnable)
  state_Detent/1 -> Aux2_Indicator/2(state_Detent), Aux1_Indicator/3(state_Detent)
  Aux2_Indicator/1(ind_Aux2) -> ind_Aux2/1
  Aux1_Indicator/1(ind_Aux1) -> ind_Aux1/1
  state_AuxEnable/1 -> Aux1_Indicator/1(state_AuxEnable)
  flag_AuxEnableFlash/1 -> Aux1_Indicator/2(flag_AuxEnableFlash)

== system MX_Gateway/MX_Gtwy_Control/PanelInterface/Backlighting ==
  dout_Light : Inport
  Backlighting : Stateflow chart  TreatAsAtomicUnit=on
  level_DisplayBacklight : Outport
  level_IconBacklight : Outport  Port=2
  wires:
  Backlighting/2(level_IconBacklight) -> level_IconBacklight/1  "level_IconBacklight"
  Backlighting/1(level_DisplayBacklight) -> level_DisplayBacklight/1  "level_DisplayBacklight"
  dout_Light/1 -> Backlighting/1(dout_Light)  "dout_Light"

== system MX_Gateway/MX_Gtwy_Control/PanelInterface/FrontLight ==
  state_LightsOutput : Inport
  dout_Lights : Inport  Port=2
  LightIndicator : Stateflow chart  TreatAsAtomicUnit=on
  ind_Light1 : Outport
  wires:
  state_LightsOutput/1 -> LightIndicator/2(state_LightsOutput)
  dout_Lights/1 -> LightIndicator/1(dout_Lights)
  LightIndicator/1(ind_Light1) -> ind_Light1/1  "ind_Light1"

== system MX_Gateway/MX_Gtwy_Control/PanelInterface/InformationDisplay ==
  msg_PanelButtonState : Inport  OutDataTypeStr=uint8
  state_ServiceClock : Inport  Port=2  OutDataTypeStr=uint8
  state_KeylessPanel : Inport  Port=3  OutDataTypeStr=uint8
  state_Engine : Inport  Port=4  OutDataTypeStr=uint8
  state_Detent : Inport  Port=5  OutDataTypeStr=uint8
  state_DetentEnable : Inport  Port=6  OutDataTypeStr=uint8
  state_CANThrottleCalDiag : Inport  Port=7  OutDataTypeStr=uint8
  state_TC : Inport  Port=8  OutDataTypeStr=uint8
  KSSDisplayState : Inport  Port=9  OutDataTypeStr=uint8
  state_MachineLock : Inport  Port=10  OutDataTypeStr=uint8
  mode_ControlChange : Inport  Port=11
  mode_LeftControl : Inport  Port=12
  mode_RightControl : Inport  Port=13
  count_GlowPlug : Inport  Port=14
  cmd_SecondAux : Inport  Port=15
  state_Key : Inport  Port=16  OutDataTypeStr=uint8
  Detect Decrease : simulink/Logic and Bit Operations/Detect Decrease  vinit=0.0  InputProcessing=Inherited  OutDataTypeStr=boolean
  Display_Type : Stateflow chart  TreatAsAtomicUnit=on
  state_InfoPrimary : Outport
  state_InfoSecondary : Outport  Port=2
  state_InfoButton : Outport  Port=3
  state_RightButton : Outport  Port=4
  state_LeftButton : Outport  Port=5
  enable_RightLeft : Outport  Port=6
  wires:
  state_Key/1 -> Display_Type/5(state_Key)  "state_Key"
  state_Engine/1 -> Display_Type/4(state_Engine)  "state_Engine"
  state_ServiceClock/1 -> Display_Type/3(state_ServiceClock)  "state_ServiceClock"
  msg_PanelButtonState/1 -> Display_Type/2(msg_PanelButtonState)  "msg_PanelButtonState"
  count_GlowPlug/1 -> Display_Type/1(count_GlowPlug)  "count_GlowPlug"
  Display_Type/6(enable_RightLeft) -> enable_RightLeft/1  "enable_RightLeft"
  state_Detent/1 -> Display_Type/6(state_Detent)
  state_DetentEnable/1 -> Display_Type/7(state_DetentEnable)
  state_CANThrottleCalDiag/1 -> Display_Type/8(state_CANThrottleCalDiag)
  state_TC/1 -> Display_Type/9(state_TC)
  Display_Type/2(state_InfoButton) -> state_InfoButton/1  "state_InfoButton"
  Display_Type/3(state_InfoSecondary) -> state_InfoSecondary/1  "state_InfoSecondary"
  Display_Type/1(state_InfoPrimary) -> state_InfoPrimary/1  "state_InfoPrimary"
  Display_Type/4(state_LeftButton) -> state_LeftButton/1  "state_LeftButton"
  Display_Type/5(state_RightButton) -> state_RightButton/1  "state_RightButton"
  KSSDisplayState/1 -> Display_Type/10(KSSDisplayState)
  state_KeylessPanel/1 -> Display_Type/11(state_KeylessPanel)
  state_MachineLock/1 -> Display_Type/12(state_MachineLock)
  cmd_SecondAux/1 -> Detect Decrease/1, Display_Type/13(cmd_SecondAux)
  Detect Decrease/1 -> Display_Type/14(fallingedge_SecondAux)
  mode_ControlChange/1 -> Display_Type/15(mode_ControlChange)
  mode_LeftControl/1 -> Display_Type/16(mode_LeftControl)
  mode_RightControl/1 -> Display_Type/17(mode_RightControl)

== system MX_Gateway/MX_Gtwy_Control/PanelInterface/Keyless_Indicators ==
  state_KSPassword : Inport
  state_KSLock : Inport  Port=2
  Keyless_Ind : Stateflow chart  TreatAsAtomicUnit=on
  ind_Lock : Outport
  ind_Unlock : Outport  Port=2
  wires:
  state_KSLock/1 -> Keyless_Ind/2(state_KSLock)  "state_KSLock"
  state_KSPassword/1 -> Keyless_Ind/1(state_KSPassword)  "state_KSPassword"
  Keyless_Ind/2(ind_Unlock) -> ind_Unlock/1  "ind_Unlock"
  Keyless_Ind/1(ind_Lock) -> ind_Lock/1  "ind_Lock"

== system MX_Gateway/MX_Gtwy_Control/PanelInterface/mod_AuxIcon ==
  state_AuxEnable : Inport
  mode_RightControl : Inport  Port=2
  Truth_Table : Truth Table  TreatAsAtomicUnit=on
  icon_Aux : Outport
  wires:
  Truth_Table/1(icon_Aux) -> icon_Aux/1
  state_AuxEnable/1 -> Truth_Table/1(state_AuxEnable)
  mode_RightControl/1 -> Truth_Table/2(mode_RightControl)

== system MX_Gateway/MX_Gtwy_Control/PanelInterface/mod_BatteryIcon ==
  state_VBattery : Inport  OutDataTypeStr=uint8
  state_Alternator : Inport  Port=2  OutDataTypeStr=uint8
  state_InfoPrimary : Inport  Port=3  OutDataTypeStr=uint8
  BatteryIcon : Stateflow chart  TreatAsAtomicUnit=on
  icon_Battery : Outport
  wires:
  BatteryIcon/1(icon_Battery) -> icon_Battery/1  "icon_Battery"
  state_VBattery/1 -> BatteryIcon/1(state_VBattery)  "state_VBattery"
  state_InfoPrimary/1 -> BatteryIcon/2(state_InfoPrimary)  "state_InfoPrimary"
  state_Alternator/1 -> BatteryIcon/3(state_Alternator)  "state_Alternator"

== system MX_Gateway/MX_Gtwy_Control/PanelInterface/mod_ECTIcon ==
  state_ECT : Inport
  WarningIcon : Stateflow chart  TreatAsAtomicUnit=on
  icon_ECT : Outport
  wires:
  WarningIcon/1(icon_ECT) -> icon_ECT/1  "icon_ECT"
  state_ECT/1 -> WarningIcon/1(state_ECT)  "state_ECT"

== system MX_Gateway/MX_Gtwy_Control/PanelInterface/mod_EngineIcon ==
  state_FuelPriOutput : Inport
  state_FuelPriRelay : Inport  Port=2
  error_FuelSec : Inport  Port=3
  state_StarterOutput : Inport  Port=4
  state_StarterRelay : Inport  Port=5
  state_GlowPlugOutput : Inport  Port=6
  state_GlowPlugRelay : Inport  Port=7
  state_EOP : Inport  Port=8
  state_ThrottlePrimary : Inport  Port=9
  state_FuelTemp : Inport  Port=10
  state_CommECU : Inport  Port=11
  state_HPMatch : Inport  Port=12
  state_EngineSpeedDerate : Inport  Port=13
  state_TurboPrime : Inport  Port=14
  lamp_Engine : Inport  Port=15
  icon_RPM : Inport  Port=16
  EngineIcon : Stateflow chart  TreatAsAtomicUnit=on
  icon_Engine : Outport
  wires:
  state_EOP/1 -> EngineIcon/6(state_EOP)  "state_EOP"
  EngineIcon/1(icon_Engine) -> icon_Engine/1  "icon_Engine"
  state_StarterRelay/1 -> EngineIcon/5(state_StarterRelay)  "state_StarterRelay"
  state_StarterOutput/1 -> EngineIcon/4(state_StarterOutput)  "state_StarterOutput"
  error_FuelSec/1 -> EngineIcon/3(error_FuelSec)  "error_FuelSec"
  state_FuelPriRelay/1 -> EngineIcon/2(state_FuelPriRelay)  "state_FuelPriRelay"
  state_FuelPriOutput/1 -> EngineIcon/1(state_FuelPriOutput)  "state_FuelPriOutput"
  state_ThrottlePrimary/1 -> EngineIcon/7(state_ThrottlePrimary)  "state_ThrottlePrimary"
  state_CommECU/1 -> EngineIcon/8(state_CommECU)  "state_CommECU"
  lamp_Engine/1 -> EngineIcon/9(lamp_Engine)  "lamp_Engine"
  state_EngineSpeedDerate/1 -> EngineIcon/10(state_EngineSpeedDerate)
  state_HPMatch/1 -> EngineIcon/11(state_HPMatch)
  icon_RPM/1 -> EngineIcon/12(icon_RPM)
  state_GlowPlugOutput/1 -> EngineIcon/13(state_GlowPlugOutput)
  state_GlowPlugRelay/1 -> EngineIcon/14(state_GlowPlugRelay)
  state_FuelTemp/1 -> EngineIcon/15(state_FuelTemp)
  state_TurboPrime/1 -> EngineIcon/16(state_TurboPrime)

== system MX_Gateway/MX_Gtwy_Control/PanelInterface/mod_FuelLevelIcon ==
  state_FuelLevel : Inport
  FuelLevel : Stateflow chart  TreatAsAtomicUnit=on
  icon_Fuel : Outport
  wires:
  FuelLevel/1(icon_Fuel) -> icon_Fuel/1  "icon_Fuel"
  state_FuelLevel/1 -> FuelLevel/1(state_FuelLevel)  "state_FuelLevel"

== system MX_Gateway/MX_Gtwy_Control/PanelInterface/mod_GeneralWarningIcon ==
  state_SensorSupply1 : Inport
  state_SensorSupply2 : Inport  Port=2
  state_Key : Inport  Port=3
  state_LoadMoment : Inport  Port=4
  state_LoadSense : Inport  Port=5
  state_OffsetPot : Inport  Port=6
  state_AuxPot : Inport  Port=7
  state_RightPot : Inport  Port=8
  state_LeftPot : Inport  Port=9
  state_SwPwrOutput : Inport  Port=10
  state_SwPwrRelay : Inport  Port=11
  state_HVACOutput : Inport  Port=12
  state_HydExchange : Inport  Port=13
  state_OffsetBase : Inport  Port=14
  state_OffsetRod : Inport  Port=15
  state_AuxBase : Inport  Port=16
  state_AuxRod : Inport  Port=17
  state_PBValveOutput : Inport  Port=18
  state_PBValveRelay : Inport  Port=19
  state_SecondAux : Inport  Port=20
  state_ConstantData : Inport  Port=21
  state_KeylessPanel : Inport  Port=22
  state_DeluxeG5 : Inport  Port=23
  state_CommThrottle : Inport  Port=24
  state_CommSecondary : Inport  Port=25
  state_CommJogShuttle : Inport  Port=26
  state_CANThrottle : Inport  Port=27
  state_CommStdPanel : Inport  Port=28
  state_CommDeluxeG55 : Inport  Port=29
  icon_Service : Inport  Port=30
  WarningIcon : Stateflow chart  TreatAsAtomicUnit=on
  icon_General : Outport
  wires:
  state_KeylessPanel/1 -> WarningIcon/7(state_KeylessPanel)  "state_KeylessPanel"
  state_ConstantData/1 -> WarningIcon/6(state_ConstantData)  "state_ConstantData"
  state_Key/1 -> WarningIcon/5(state_Key)  "state_Key"
  state_SensorSupply2/1 -> WarningIcon/4(state_SensorSupply2)  "state_SensorSupply2"
  state_SensorSupply1/1 -> WarningIcon/3(state_SensorSupply1)  "state_SensorSupply1"
  state_SwPwrRelay/1 -> WarningIcon/2(state_SwPwrRelay)  "state_SwPwrRelay"
  state_SwPwrOutput/1 -> WarningIcon/1(state_SwPwrOutput)  "state_SwPwrOutput"
  WarningIcon/1(icon_General) -> icon_General/1  "icon_General"
  state_DeluxeG5/1 -> WarningIcon/8(state_DeluxeG5)  "state_DeluxeG5"
  state_HVACOutput/1 -> WarningIcon/9(state_HVACOutput)  "state_HVACOutput"
  state_CommThrottle/1 -> WarningIcon/10(state_CommThrottle)
  state_HydExchange/1 -> WarningIcon/11(state_HydExchange)
  state_LoadMoment/1 -> WarningIcon/12(state_LoadMoment)
  state_LoadSense/1 -> WarningIcon/13(state_LoadSense)
  state_OffsetBase/1 -> WarningIcon/15(state_OffsetBase)
  state_OffsetPot/1 -> WarningIcon/14(state_OffsetPot)
  state_OffsetRod/1 -> WarningIcon/16(state_OffsetRod)
  state_CommSecondary/1 -> WarningIcon/20(state_CommSecondary)
  state_CommJogShuttle/1 -> WarningIcon/21(state_CommJogShuttle)
  state_AuxPot/1 -> WarningIcon/17(state_AuxPot)
  state_AuxBase/1 -> WarningIcon/18(state_AuxBase)
  state_AuxRod/1 -> WarningIcon/19(state_AuxRod)
  icon_Service/1 -> WarningIcon/24(icon_Service)
  state_CANThrottle/1 -> WarningIcon/22(state_CANThrottle)
  state_PBValveOutput/1 -> WarningIcon/25(state_PBValveOutput)
  state_PBValveRelay/1 -> WarningIcon/26(state_PBValveRelay)
  state_CommStdPanel/1 -> WarningIcon/23(state_CommStdPanel)
  state_CommDeluxeG55/1 -> WarningIcon/27(state_CommDeluxeG55)
  state_SecondAux/1 -> WarningIcon/28(state_SecondAux)
  state_RightPot/1 -> WarningIcon/29(state_RightPot)
  state_LeftPot/1 -> WarningIcon/30(state_LeftPot)

== system MX_Gateway/MX_Gtwy_Control/PanelInterface/mod_GlowPlugIcon ==
  dout_GlowPlug : Inport
  state_GlowPlugOutput : Inport  Port=2
  state_GlowPlugRelay : Inport  Port=3
  ecu_GlowPlug : Inport  Port=4
  state_Engine : Inport  Port=5
  GlowPlugIcon : Stateflow chart  TreatAsAtomicUnit=on
  icon_GlowPlug : Outport
  wires:
  GlowPlugIcon/1(icon_GlowPlug) -> icon_GlowPlug/1  "icon_GlowPlug"
  state_GlowPlugRelay/1 -> GlowPlugIcon/3(state_GlowPlugRelay)  "state_GlowPlugRelay"
  state_GlowPlugOutput/1 -> GlowPlugIcon/2(state_GlowPlugOutput)  "state_GlowPlugOutput"
  dout_GlowPlug/1 -> GlowPlugIcon/1(dout_GlowPlug)  "dout_GlowPlug"
  ecu_GlowPlug/1 -> GlowPlugIcon/4(ecu_GlowPlug)  "ecu_GlowPlug"
  state_Engine/1 -> GlowPlugIcon/5(state_Engine)

== system MX_Gateway/MX_Gtwy_Control/PanelInterface/mod_HourMeterIcon ==
  state_InfoPrimary : Inport  OutDataTypeStr=uint8
  state_InfoSecondary : Inport  Port=2  OutDataTypeStr=uint8
  Truth_Table : Truth Table  TreatAsAtomicUnit=on
  icon_Hourmeter : Outport
  wires:
  state_InfoSecondary/1 -> Truth_Table/2(state_InfoSecondary)  "state_InfoSecondary"
  Truth_Table/1(icon_Hourmeter) -> icon_Hourmeter/1  "icon_Hourmeter"
  state_InfoPrimary/1 -> Truth_Table/1(state_InfoPrimary)  "state_InfoPrimary"

== system MX_Gateway/MX_Gtwy_Control/PanelInterface/mod_HydraulicIcon ==
  state_HOT : Inport
  HydraulicIcon : Stateflow chart  TreatAsAtomicUnit=on
  icon_Hydraulic : Outport
  wires:
  HydraulicIcon/1(icon_Hydraulic) -> icon_Hydraulic/1  "icon_Hydraulic"
  state_HOT/1 -> HydraulicIcon/1(state_HOT)  "state_HOT"

== system MX_Gateway/MX_Gtwy_Control/PanelInterface/mod_JobClock1Icon ==
  state_InfoPrimary : Inport
  Truth_Table : Truth Table  TreatAsAtomicUnit=on
  icon_JobClock1 : Outport
  wires:
  Truth_Table/1(y) -> icon_JobClock1/1  "icon_JobClock1"
  state_InfoPrimary/1 -> Truth_Table/1(u)  "state_InfoPrimary"

== system MX_Gateway/MX_Gtwy_Control/PanelInterface/mod_JobClock2Icon ==
  state_InfoPrimary : Inport
  Truth_Table : Truth Table  TreatAsAtomicUnit=on
  icon_JobClock2 : Outport
  wires:
  Truth_Table/1(y) -> icon_JobClock2/1  "icon_JobClock2"
  state_InfoPrimary/1 -> Truth_Table/1(u)  "state_InfoPrimary"

== system MX_Gateway/MX_Gtwy_Control/PanelInterface/mod_LockoutIcon ==
  state_Console : Inport
  state_ConsoleTest : Inport  Port=2
  state_HydEnable : Inport  Port=3
  state_HydBypass : Inport  Port=4
  LockoutIcons : Stateflow chart  TreatAsAtomicUnit=on
  icon_Lockout : Outport
  wires:
  state_HydEnable/1 -> LockoutIcons/3(state_HydEnable)
  LockoutIcons/1(icon_Lockout) -> icon_Lockout/1
  state_ConsoleTest/1 -> LockoutIcons/2(state_ConsoleTest)
  state_Console/1 -> LockoutIcons/1(state_Console)
  state_HydBypass/1 -> LockoutIcons/4(state_HydBypass)

== system MX_Gateway/MX_Gtwy_Control/PanelInterface/mod_RPMIcon ==
  state_InfoPrimary : Inport
  state_EngineSpeed : Inport  Port=2
  RPM_ICON : Stateflow chart  TreatAsAtomicUnit=on
  icon_RPM : Outport
  wires:
  RPM_ICON/1(icon_RPM) -> icon_RPM/1  "icon_RPM"
  state_EngineSpeed/1 -> RPM_ICON/2(state_EngineSpeed)  "state_EngineSpeed"
  state_InfoPrimary/1 -> RPM_ICON/1(state_InfoPrimary)  "state_InfoPrimary"

== system MX_Gateway/MX_Gtwy_Control/PanelInterface/mod_SeatBeltIcon ==
  Chart : Stateflow chart  TreatAsAtomicUnit=on
  icon_Seatbelt : Outport
  wires:
  Chart/1(icon_Seatbelt) -> icon_Seatbelt/1  "icon_Seatbelt"

== system MX_Gateway/MX_Gtwy_Control/PanelInterface/mod_ServiceClockIcon ==
  state_InfoSecondary : Inport
  state_AirFilter : Inport  Port=2
  state_HCFilter1 : Inport  Port=3
  state_InfoPrimary : Inport  Port=4
  Truth_Table : Truth Table  TreatAsAtomicUnit=on
  icon_Service : Outport
  wires:
  state_InfoPrimary/1 -> Truth_Table/4(state_InfoPrimary)  "state_InfoPrimary"
  state_HCFilter1/1 -> Truth_Table/3(state_HCFilter1)  "state_HCFilter1"
  state_AirFilter/1 -> Truth_Table/2(state_AirFilter)  "state_AirFilter"
  state_InfoSecondary/1 -> Truth_Table/1(state_InfoSecondary)  "state_InfoSecondary"
  Truth_Table/1(icon_Service) -> icon_Service/1  "icon_Service"

== system MX_Gateway/MX_Gtwy_Control/PanelInterface/mod_TwoSpeedIcon ==
  state_TwoSpeed : Inport
  state_TwoSpeedIcon : Inport  Port=2
  RabbitIcon : Stateflow chart  TreatAsAtomicUnit=on
  icon_Rabbit : Outport
  wires:
  RabbitIcon/1(icon_Rabbit) -> icon_Rabbit/1
  state_TwoSpeed/1 -> RabbitIcon/1(state_TwoSpeed)
  state_TwoSpeedIcon/1 -> RabbitIcon/2(state_TwoSpeedIcon)
